simulink model "BaseEngineController_A02_bak"  (Simulink 7.7)
settings: Solver=FixedStepDiscrete  StartTime=0.0  StopTime=10.0  RelTol=1e-3
PreLoadFcn:
  | try, eval([bdroot '_setup']); catch, eval('model_setup', ''); end
InitFcn:
  | try, eval([bdroot '_setup']); catch, eval('model_setup', ''); end
undefined symbols (not defined in the file or in its folder): ECU555, High, motohawk_build_version, motohawk_get_faults, motohawk_trunc_name, motohawk_vardec_config_group, motohawk_vardec_path, True
libraries: MotoHawk_lib x1145, AutoDoc_lib x786, motohawk_control_primitives_lib x114, motohawk_engine_lib x19, motohawk_annotations_lib x12, simulink x11, simulink_extras x1
(part 23 of 23)

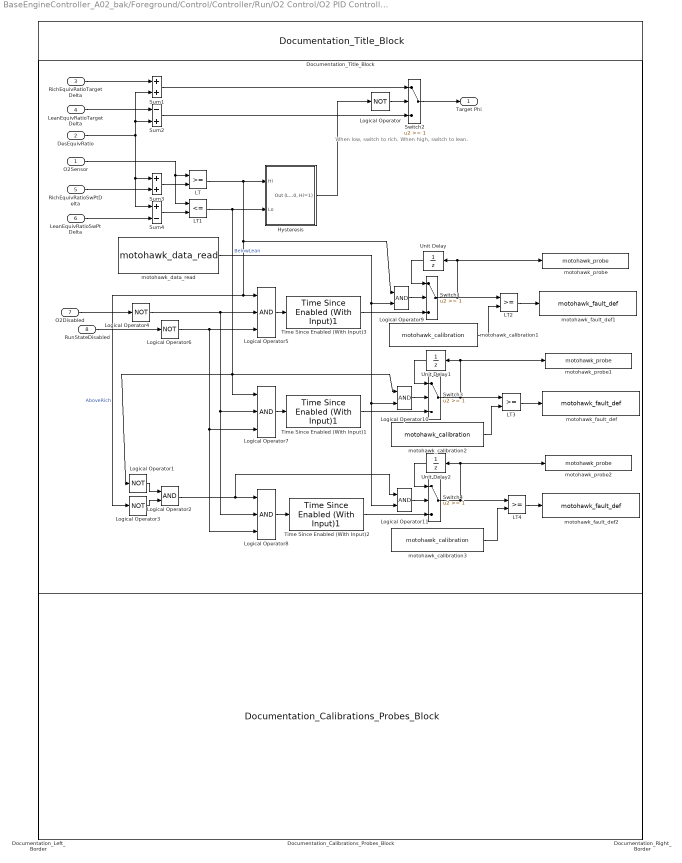
== system BaseEngineController_A02_bak/Foreground/Control/Controller/Run/O2 Control/O2 PID Controller/Equivalence Ratio Perturbation ==
  note: When low, switch to rich.
      | When high, switch to lean.
  O2Sensor : Inport
  DesEquivRatio : Inport  Port=2
  RichEquivRatioTargetDelta : Inport  Port=3
  LeanEquivRatioTargetDelta : Inport  Port=4
  RichEquivRatioSwPtDelta : Inport  Port=5
  LeanEquivRatioSwPtDelta : Inport  Port=6
  O2Disabled : Inport  Port=7
  RunStateDisabled : Inport  Port=8
  Documentation_Calibrations_Probes_Block : AutoDoc_lib/Documentation_Calibrations_Probes_Block
  Documentation_Left_Border : AutoDoc_lib/Documentation_Left_Border  layout=Portrait
  Documentation_Right_Border : AutoDoc_lib/Documentation_Right_Border  layout=Portrait
  Documentation_Title_Block : AutoDoc_lib/Documentation_Title_Block
  Hysteresis : Subsystem
  LT{,2..4} (4 alike) : RelationalOperator  ZeroCross=off  InputSameDT=on
  LT1 : RelationalOperator  Operator=<=  ZeroCross=off  InputSameDT=on
  Logical Operator{,1,3..4,6} (5 alike) : Logic  Operator=NOT
  Logical Operator{2,9..11} (4 alike) : Logic
  Logical Operator5 : Logic  Inputs=3
  Logical Operator7 : Logic  Inputs=3
  Logical Operator8 : Logic  Inputs=3
  Sum1 : Sum  SaturateOnIntegerOverflow=on
  Sum2 : Sum  Inputs=-+  SaturateOnIntegerOverflow=on
  Sum3 : Sum  SaturateOnIntegerOverflow=on
  Sum4 : Sum  Inputs=+-  SaturateOnIntegerOverflow=on
  Switch{1..4} (4 alike) : Switch  Threshold=1  ZeroCross=off  InputSameDT=on
  Time Since Enabled (With Input)1 : motohawk_control_primitives_lib/Time Since Enabled (With Input)1  SystemSampleTime=-1  nam='O2LeanTimer'  samp_time=-1  init=0  upperlim=16000
  Time Since Enabled (With Input)2 : motohawk_control_primitives_lib/Time Since Enabled (With Input)1  SystemSampleTime=-1  nam='O2ActiveTimer'  samp_time=-1  init=0  upperlim=16000
  Time Since Enabled (With Input)3 : motohawk_control_primitives_lib/Time Since Enabled (With Input)1  SystemSampleTime=-1  nam='O2RichTimer'  samp_time=-1  init=0  upperlim=16000
  Unit Delay : UnitDelay  SampleTime=-1
  Unit Delay1 : UnitDelay  SampleTime=-1
  Unit Delay2 : UnitDelay  SampleTime=-1
  motohawk_calibration1 : MotoHawk_lib/Calibration & Probing Blocks/motohawk_calibration  nam='O2RichTimeThresh'  val=40  storage=Calibration  show_more=on  name_source=Use Parameter  data_type=Inherit from 'Default Value'  read_level=1  write_level=1  ovr_cals=on  view_as=Number  enum_struct={'Disabled', 'Enabled'}  vector_style=Wide Row  use_help_units=on  help='Time limit the O2 feedback can sit abovethe rich switch point before flagging O2FailedRich fault'  units='s'  use_header_enum=off  row_header_enum={}  col_header_enum={}  use_min_max=off  min_val=-inf  max_val=inf  use_precision=off  precision=''  gain=1  offset=0  exp=1  use_mototune_group=on  mototune_group='Diagnostics & Protection | Fault Thresholds'
  motohawk_calibration2 : MotoHawk_lib/Calibration & Probing Blocks/motohawk_calibration  nam='O2LeanTimeThresh'  val=10  storage=Calibration  show_more=on  name_source=Use Parameter  data_type=Inherit from 'Default Value'  read_level=1  write_level=1  ovr_cals=on  view_as=Number  enum_struct={'Disabled', 'Enabled'}  vector_style=Wide Row  use_help_units=on  help='Time limit the O2 feedback can sit below the lean switch point before flagging O2FailedLean fault'  units='s'  use_header_enum=off  row_header_enum={}  col_header_enum={}  use_min_max=off  min_val=-inf  max_val=inf  use_precision=off  precision=''  gain=1  offset=0  exp=1  use_mototune_group=on  mototune_group='Diagnostics & Protection | Fault Thresholds'
  motohawk_calibration3 : MotoHawk_lib/Calibration & Probing Blocks/motohawk_calibration  nam='O2ActiveTimeThresh'  val=40  storage=Calibration  show_more=on  name_source=Use Parameter  data_type=Inherit from 'Default Value'  read_level=1  write_level=1  ovr_cals=on  view_as=Number  enum_struct={'Disabled', 'Enabled'}  vector_style=Wide Row  use_help_units=on  help='Time limit O2 feedback can sit between rich and lean switch points before flagging O2NotActive fault'  units='s'  use_header_enum=off  row_header_enum={}  col_header_enum={}  use_min_max=off  min_val=-inf  max_val=inf  use_precision=off  precision=''  gain=1  offset=0  exp=1  use_mototune_group=on  mototune_group='Diagnostics & Protection | Fault Thresholds'
  motohawk_data_read : MotoHawk_lib/Data Storage Blocks/motohawk_data_read  source=Lookup By Name  structure=Scalar  operation=Read scalar from location by index  sz_vec=4  sz_row=2  sz_col=3  typ=boolean  nam='DisableO2FaultAction'  checkNull=on  struct_inst_name='Struct1'  struct_nam='MyStruct'
  motohawk_fault_def : MotoHawk_lib/Fault Management Blocks/motohawk_fault_def  nam='O2FailedLean'  mode=Enabled  id=[]  x=25  y=50  input_suspected=on  indeterminate=off  increment_xy=on  downsample_count=1  ovr_cals=on  ovr_actions=on  show_actions=off  action1_name=''  action1_cond=(None)  action2_name=''  action2_cond=(None)  action3_name=''  action3_cond=(None)  action4_name=''  action4_cond=(None)
  motohawk_fault_def1 : MotoHawk_lib/Fault Management Blocks/motohawk_fault_def  nam='O2FailedRich'  mode=Enabled  id=[]  x=25  y=50  input_suspected=on  indeterminate=off  increment_xy=on  downsample_count=1  ovr_cals=on  ovr_actions=on  show_actions=off  action1_name=''  action1_cond=(None)  action2_name=''  action2_cond=(None)  action3_name=''  action3_cond=(None)  action4_name=''  action4_cond=(None)
  motohawk_fault_def2 : MotoHawk_lib/Fault Management Blocks/motohawk_fault_def  nam='O2NotActive'  mode=Enabled  id=[]  x=25  y=50  input_suspected=on  indeterminate=off  increment_xy=on  downsample_count=1  ovr_cals=on  ovr_actions=on  show_actions=off  action1_name=''  action1_cond=(None)  action2_name=''  action2_cond=(None)  action3_name=''  action3_cond=(None)  action4_name=''  action4_cond=(None)
  motohawk_probe : MotoHawk_lib/Calibration & Probing Blocks/motohawk_probe  nam='O2RichTime'  show_more=on  name_source=Use Parameter  read_level=1  view_as=Number  enum_struct={'Disabled', 'Enabled'}  vector_style=Wide Row  use_help_units=off  help=''  units=''  use_header_enum=off  row_header_enum={}  col_header_enum={}  use_precision=off  precision=''  gain=1  offset=0  exp=1  use_mototune_group=on  mototune_group='Diagnostics & Protection | Fault Thresholds'
  motohawk_probe1 : MotoHawk_lib/Calibration & Probing Blocks/motohawk_probe  nam='O2LeanTime'  show_more=on  name_source=Use Parameter  read_level=1  view_as=Number  enum_struct={'Disabled', 'Enabled'}  vector_style=Wide Row  use_help_units=off  help=''  units=''  use_header_enum=off  row_header_enum={}  col_header_enum={}  use_precision=off  precision=''  gain=1  offset=0  exp=1  use_mototune_group=on  mototune_group='Diagnostics & Protection | Fault Thresholds'
  motohawk_probe2 : MotoHawk_lib/Calibration & Probing Blocks/motohawk_probe  nam='O2NotActiveTime'  show_more=on  name_source=Use Parameter  read_level=1  view_as=Number  enum_struct={'Disabled', 'Enabled'}  vector_style=Wide Row  use_help_units=off  help=''  units=''  use_header_enum=off  row_header_enum={}  col_header_enum={}  use_precision=off  precision=''  gain=1  offset=0  exp=1  use_mototune_group=on  mototune_group='Diagnostics & Protection | Fault Thresholds'
  Target Phi : Outport
  wires:
  Sum3/1 -> LT/2
  Sum4/1 -> LT1/2
  Switch2/1 -> Target Phi/1
  DesEquivRatio/1 -> Sum1/2, Sum2/2, Sum4/1, Sum3/1
  RichEquivRatioSwPtDelta/1 -> Sum3/2
  LeanEquivRatioSwPtDelta/1 -> Sum4/2
  Sum2/1 -> Switch2/3
  Sum1/1 -> Switch2/1
  LeanEquivRatioTargetDelta/1 -> Sum2/1
  RichEquivRatioTargetDelta/1 -> Sum1/1
  LT/1 -> Hysteresis/1(Hi), Logical Operator5/1, Logical Operator3/1, Logical Operator9/1  "AboveRich"
  LT1/1 -> Hysteresis/2(Lo), Logical Operator1/1, Logical Operator7/1, Logical Operator10/1  "BelowLean"
  Hysteresis/1("Out (Lo=0, Hi=1)") -> Logical Operator/1
  O2Sensor/1 -> LT1/1, LT/1
  Logical Operator/1 -> Switch2/2
  motohawk_calibration1/1 -> LT2/2
  LT2/1 -> motohawk_fault_def1/1
  LT3/1 -> motohawk_fault_def/1
  motohawk_calibration2/1 -> LT3/2
  LT4/1 -> motohawk_fault_def2/1
  motohawk_calibration3/1 -> LT4/2
  Logical Operator1/1 -> Logical Operator2/1
  Logical Operator3/1 -> Logical Operator2/2
  Logical Operator4/1 -> Logical Operator5/2, Logical Operator7/2, Logical Operator8/2
  O2Disabled/1 -> Logical Operator4/1
  RunStateDisabled/1 -> Logical Operator6/1
  Logical Operator6/1 -> Logical Operator5/3, Logical Operator7/3, Logical Operator8/3
  Logical Operator2/1 -> Logical Operator8/1, Logical Operator11/1
  Logical Operator5/1 -> Time Since Enabled (With Input)3/1
  Logical Operator7/1 -> Time Since Enabled (With Input)1/1
  Logical Operator8/1 -> Time Since Enabled (With Input)2/1
  Unit Delay/1 -> Switch1/1
  Time Since Enabled (With Input)3/1 -> Switch1/3
  Switch1/1 -> LT2/1, Unit Delay/1, motohawk_probe/1
  Logical Operator9/1 -> Switch1/2
  motohawk_data_read/1 -> Logical Operator9/2, Logical Operator10/2, Logical Operator11/2
  Unit Delay1/1 -> Switch3/1
  Switch3/1 -> LT3/1, Unit Delay1/1, motohawk_probe1/1
  Logical Operator10/1 -> Switch3/2
  Time Since Enabled (With Input)1/1 -> Switch3/3
  Unit Delay2/1 -> Switch4/1
  Switch4/1 -> LT4/1, Unit Delay2/1, motohawk_probe2/1
  Logical Operator11/1 -> Switch4/2
  Time Since Enabled (With Input)2/1 -> Switch4/3

== system BaseEngineController_A02_bak/Foreground/Control/Diagnostics/Service Intervals/Run Timers/hour meter/motohawk_override_abs ==
  note: MotoHawk Absolute Override
  In1 : Inport
  Data Type Conversion : DataTypeConversion
  Gain : Gain  Gain=3600
  If : If  IfExpression=u1 ~= 0
  Merge : Merge
  NewValue : Subsystem  TreatAsAtomicUnit=on
  OldValue : Subsystem  TreatAsAtomicUnit=on
  new_value : MotoHawk_lib/Calibration & Probing Blocks/motohawk_calibration  nam=[parent_name '_new']  val=0  storage=Display  show_more=on  name_source=Use Parameter  data_type=Inherit via back propagation  read_level=1  write_level=1  ovr_cals=on  view_as=Number  enum_struct=enum_struct  vector_style=Wide Row  use_help_units=on  help=parent_help  units=parent_units  use_header_enum=off  row_header_enum={}  col_header_enum={}  use_min_max=on  min_val=parent_min  max_val=parent_max  use_precision=on  precision=parent_precision  gain=parent_gain  offset=parent_offset  exp=parent_exp  use_mototune_group=on  mototune_group=group
  override_enable : MotoHawk_lib/Calibration & Probing Blocks/motohawk_calibration  nam=[parent_name '_ovr']  val=false  storage=Display  show_more=on  name_source=Use Parameter  data_type=boolean  read_level=1  write_level=1  ovr_cals=on  view_as=Enumeration  enum_struct={'Pass-Through', 'Override'}  vector_style=Wide Row  use_help_units=off  help=''  units=''  use_header_enum=off  row_header_enum={}  col_header_enum={}  use_min_max=off  min_val=-inf  max_val=inf  use_precision=off  precision=''  gain=1  offset=0  exp=1  use_mototune_group=on  mototune_group=group
  Out1 : Outport
  wires:
  In1/1 -> OldValue/1(In1)
  Merge/1 -> Out1/1
  NewValue/1(Out1) -> Merge/1
  OldValue/1(Out1) -> Merge/2
  override_enable/1 -> If/1
  If/1 -> NewValue/Action
  If/2 -> OldValue/Action
  new_value/1 -> Gain/1
  Data Type Conversion/1 -> NewValue/1(In1)
  Gain/1 -> Data Type Conversion/1

== system BaseEngineController_A02_bak/Foreground/Control/VirtualSensors/LoadBasedCalcs/Normalized Effective Combustion Chamber Temperature/Integrate Offset To ECT/Integrator ==
  note: Discrete Integrator,
      | with output and state saturation.
  In : Inport
  Rst : Inport  Port=2
  IC : Inport  Port=3
  Min : Inport  Port=4
  Max : Inport  Port=5
  Data Type Conversion : DataTypeConversion  RndMeth=Zero
  Product : Product  SaturateOnIntegerOverflow=on  InputSameDT=on  OutDataTypeStr=Inherit: Same as first input
  Saturation : Subsystem  mask(Saturation){}
      mask help: Saturation block.
      mask Display:
      | patch([0 0 1 1], [0 0.075 0.075 0], [230/255 0/255 0/255])
      | patch([0 0 1 1], [0.025 0.05 0.05 0.025], [150/255 0/255 0/255])
      | patch([0 0 1 1], [0 0.025 0.025 0], [0/255 0/255 0/255])
      | 
      | plot([.2 .4 .6 .8], [.2 .2 .9 .9])
  Sum : Sum  Inputs=++|  SaturateOnIntegerOverflow=on  InputSameDT=on
  Switch1 : Switch  Threshold=1  ZeroCross=off  SaturateOnIntegerOverflow=on  InputSameDT=on
  Unit Delay : UnitDelay  SampleTime=-1
  motohawk_delta_time1 : MotoHawk_lib/Extra Development Blocks/motohawk_delta_time  samp_time=-1  init_val=0  mode=Seconds (double)
  Out : Outport
  wires:
  Saturation/1(out) -> Unit Delay/1
  Sum/1 -> Saturation/1(in)
  Switch1/1 -> Sum/1, Out/1
  motohawk_delta_time1/1 -> Product/2
  In/1 -> Product/1
  Product/1 -> Sum/2
  Unit Delay/1 -> Switch1/3
  Rst/1 -> Data Type Conversion/1
  Data Type Conversion/1 -> Switch1/2
  Min/1 -> Saturation/2(min)
  Max/1 -> Saturation/3(max)
  IC/1 -> Switch1/1

== system BaseEngineController_A02_bak/Foreground/Control/Controller/Run/Fuel System Manager/Fuel Calculation/Calculate Fuel Flow/Calculate A/F Ratio ==
  Ethanol_xx [%] : Inport
  " " : Constant  Value=1.0
  Add : Sum
  Add1 : Sum  Inputs=+-
  Documentation_Calibrations_Probes_Block : AutoDoc_lib/Documentation_Calibrations_Probes_Block
  Documentation_Left_Border : AutoDoc_lib/Documentation_Left_Border  layout=Portrait
  Documentation_Right_Border : AutoDoc_lib/Documentation_Right_Border  layout=Portrait
  Documentation_Title_Block : AutoDoc_lib/Documentation_Title_Block
  Gain : Gain  Gain=.01
  Product1 : Product  RndMeth=Zero
  Product2 : Product  RndMeth=Zero
  motohawk_calibration2 : MotoHawk_lib/Calibration & Probing Blocks/motohawk_calibration  nam='AF_Gasoline'  val=14.6  storage=Calibration  show_more=on  name_source=Use Parameter  data_type=Inherit from 'Default Value'  read_level=1  write_level=1  ovr_cals=on  view_as=Number  enum_struct={'Disabled', 'Enabled'}  vector_style=Wide Row  use_help_units=on  help='Stoichiometric ratio for gasoline'  units='mult'  use_header_enum=off  row_header_enum={}  col_header_enum={}  use_min_max=on  min_val=5  max_val=30  use_precision=off  precision=''  gain=1  offset=0  exp=1  use_mototune_group=on  mototune_group=motohawk_vardec_path(gcb)
  motohawk_calibration3 : MotoHawk_lib/Calibration & Probing Blocks/motohawk_calibration  nam='AF_Ethanol'  val=9.0  storage=Calibration  show_more=on  name_source=Use Parameter  data_type=Inherit from 'Default Value'  read_level=1  write_level=1  ovr_cals=on  view_as=Number  enum_struct={'Disabled', 'Enabled'}  vector_style=Wide Row  use_help_units=on  help='Stoichiometric ratio for ethanol'  units='mult'  use_header_enum=off  row_header_enum={}  col_header_enum={}  use_min_max=on  min_val=5  max_val=30  use_precision=off  precision=''  gain=1  offset=0  exp=1  use_mototune_group=on  mototune_group=motohawk_vardec_path(gcb)
  Desired A/F Ratio : Outport
  wires:
  motohawk_calibration3/1 -> Product2/1
  motohawk_calibration2/1 -> Product1/1
  Product1/1 -> Add/1
  Product2/1 -> Add/2
  Add/1 -> Desired A/F Ratio/1
  Gain/1 -> Product2/2, Add1/2
  Add1/1 -> Product1/2
  " "/1 -> Add1/1
  Ethanol_xx [%]/1 -> Gain/1

== system BaseEngineController_A02_bak/Foreground/Control/Controller/Run/Fuel System Manager/Injector Manager/Delta TPS Transient Fueling/ECT Transient Fueling ==
  ECTIdx : Inport
  Enable : EnablePort
  Documentation_Calibrations_Probes_Block : AutoDoc_lib/Documentation_Calibrations_Probes_Block
  Documentation_Left_Border : AutoDoc_lib/Documentation_Left_Border  layout=Portrait
  Documentation_Right_Border : AutoDoc_lib/Documentation_Right_Border  layout=Portrait
  Documentation_Title_Block : AutoDoc_lib/Documentation_Title_Block
  motohawk_interpolation_1d : MotoHawk_lib/Lookup Tables/motohawk_interpolation_1d  nam='ECTTransFuelMult'  table_data=[1 1 1 1 1]  show_more=on  data_type=Inherit from 'Table Data'  storage=NonAdaptive  read_level=1  write_level=1  ovr_cals=on  use_help=on  help="'Transient fuel multiplier based on ECT.  Only engaged during transient fueling'"  units=''  use_min_max=off  min_val=-inf  max_val=inf  use_precision=off  precision=''  gain=1  offset=0  exp=1  use_links=off  prelookup_block=''  output_name=''  use_mototune_group=on  mototune_group=motohawk_vardec_path(gcb)
  Mult : Outport  OutputWhenDisabled=reset  InitialOutput=1
  wires:
  motohawk_interpolation_1d/1 -> Mult/1
  ECTIdx/1 -> motohawk_interpolation_1d/1

== system BaseEngineController_A02_bak/Foreground/Control/Controller/Run/Fuel System Manager/Injector Manager/Tau-X Transient Fueling/Enabled Tau-X Algorithm ==
  note: Calculate fuel vaporization factor.
      | Fuel evaporation is linear with respect to this term.
      | FilmTemp / ln(ManifoldPressure)
  MAF [g/s] : Inport
  IAT [degC] : Inport  Port=2
  ECT [degC] : Inport  Port=3
  MAP [kPa] : Inport  Port=4
  Fdes [g/s] : Inport  Port=5
  Action Port : ActionPort  InitializeStates=reset  ActionType=then
  Add : Sum
  Add1 : Sum
  Cals_and_Probes_Page : AutoDoc_lib/Cals_and_Probes_Page  SystemSampleTime=-1
  Constant : Constant  Value=273.15
  Documentation_Calibrations_Probes_Block : AutoDoc_lib/Documentation_Calibrations_Probes_Block
  Documentation_Left_Border : AutoDoc_lib/Documentation_Left_Border  layout=Portrait
  Documentation_Right_Border : AutoDoc_lib/Documentation_Right_Border  layout=Portrait
  Documentation_Title_Block : AutoDoc_lib/Documentation_Title_Block
  Film Temperature Average of ECT and IAT : Fcn  Expr=(u(1)*u(3)/100 + u(2)*(1-u(3)/100))
  Math Function : Math  Operator=log  SaturateOnIntegerOverflow=on
  Mux : Mux  Inputs=3
  Product : Product  Inputs=*/  SaturateOnIntegerOverflow=on  InputSameDT=on  OutDataTypeStr=Inherit: Same as first input
  Saturation : Saturate  UpperLimit=inf  LowerLimit=0
  Saturation1 : Saturate  UpperLimit=inf  LowerLimit=.0001
  Saturation2 : Saturate  UpperLimit=inf  LowerLimit=.0001
  Tau-X Fuel Model : Subsystem  mask{samp_time=-1}
      mask prompts: samp_time "Sample Time"
      mask Display:
      | patch([0 0 1 1], 0.5*[0 0.075 0.075 0], [230/255 0/255 0/255])
      | patch([0 0 1 1], 0.5*[0.025 0.05 0.05 0.025], [150/255 0/255 0/255])
      | patch([0 0 1 1], 0.5*[0 0.025 0.025 0], [0/255 0/255 0/255])
      | 
      | disp('TRANSIENT FUELING\nTAU-X MODEL');
  Zero-Order Hold : ZeroOrderHold  SampleTime=-1
  motohawk_calibration1 : MotoHawk_lib/Calibration & Probing Blocks/motohawk_calibration  nam='FuelVaporFactorBias'  val=50  storage=Calibration  show_more=on  name_source=Use Parameter  data_type=Inherit from 'Default Value'  read_level=1  write_level=1  ovr_cals=on  view_as=Number  enum_struct={'Disabled', 'Enabled'}  vector_style=Wide Row  use_help_units=on  help="'Percent bias between contribution of IAT and ECT to FuelVaporFactor.  100% is all IAT.'"  units='%'  use_header_enum=off  row_header_enum={}  col_header_enum={}  use_min_max=on  min_val=0  max_val=100  use_precision=off  precision=''  gain=1  offset=0  exp=1  use_mototune_group=on  mototune_group=motohawk_vardec_path(gcb)
  motohawk_calibration2 : MotoHawk_lib/Calibration & Probing Blocks/motohawk_calibration  nam='TransientFuelMaxPuddle'  val=1000  storage=Calibration  show_more=on  name_source=Use Parameter  data_type=Inherit from 'Default Value'  read_level=1  write_level=1  ovr_cals=on  view_as=Number  enum_struct={'Disabled', 'Enabled'}  vector_style=Wide Row  use_help_units=on  help='Maximum fuel puddle size'  units='mg'  use_header_enum=off  row_header_enum={}  col_header_enum={}  use_min_max=on  min_val=0  max_val=inf  use_precision=off  precision=''  gain=1  offset=0  exp=1  use_mototune_group=on  mototune_group=motohawk_vardec_path(gcb)
  motohawk_calibration3 : MotoHawk_lib/Calibration & Probing Blocks/motohawk_calibration  nam='TransientFuelInitPuddle'  val=20  storage=Calibration  show_more=on  name_source=Use Parameter  data_type=Inherit from 'Default Value'  read_level=1  write_level=1  ovr_cals=on  view_as=Number  enum_struct={'Disabled', 'Enabled'}  vector_style=Wide Row  use_help_units=on  help='Initial fuel puddle size'  units='mg'  use_header_enum=off  row_header_enum={}  col_header_enum={}  use_min_max=on  min_val=0  max_val=inf  use_precision=off  precision=''  gain=1  offset=0  exp=1  use_mototune_group=on  mototune_group=motohawk_vardec_path(gcb)
  motohawk_interpolation_2d1 : MotoHawk_lib/Lookup Tables/motohawk_interpolation_2d  nam='TransientFuelTau'  table_data=[1 0.87 0.74 0.61 0.5;0.87 0.78 0.65 0.51 0.4;0.74 0.65 0.56 0.43 0.3;0.61 0.51 0.43 0.36 0.2;0.5 0.4 0.3 0.2 0.1]  show_more=on  data_type=Inherit from 'Table Data'  storage=NonAdaptive  read_level=1  write_level=1  ovr_cals=on  use_help=on  help='Fuel evaporation time constant'  units='sec'  use_min_max=on  min_val=0  max_val=inf  use_precision=off  precision=''  gain=1  offset=0  exp=1  use_links=off  row_prelookup_block=''  col_prelookup_block=''  output_name=''  use_mototune_group=on  mototune_group=motohawk_vardec_path(gcb)
  motohawk_interpolation_2d2 : MotoHawk_lib/Lookup Tables/motohawk_interpolation_2d  nam='TransientFuelX'  table_data=[0.6016 0.5234 0.4531 0.375 0.2969;0.5547 0.4844 0.4141 0.3438 0.2734;0.5 0.4375 0.3828 0.3125 0.25;0.4531 0.3984 0.3438 0.2813 0.2266;0.3984 0.3516 0.3047 0.25 0.2031]  show_more=on  data_type=Inherit from 'Table Data'  storage=NonAdaptive  read_level=1  write_level=1  ovr_cals=on  use_help=on  help='Fraction of injected fuel to enter the puddle'  units='frac'  use_min_max=on  min_val=0  max_val=.999  use_precision=off  precision=''  gain=1  offset=0  exp=1  use_links=off  row_prelookup_block=''  col_prelookup_block=''  output_name=''  use_mototune_group=on  mototune_group=motohawk_vardec_path(gcb)
  motohawk_prelookup2 : MotoHawk_lib/Lookup Tables/motohawk_prelookup  nam='FuelVapFactor'  breakpoint_data=[-5 2.5 10 17.5 25]  show_more=on  data_type=Inherit from 'Table Data'  storage=Constant  read_level=1  write_level=1  ovr_cals=on  use_help=on  help='Breakpoints for fuel vaporization factor'  units='degC/log(kPa)'  use_min_max=off  min_val=-inf  max_val=inf  use_precision=off  precision=''  gain=1  offset=0  exp=1  use_links=on  input_name=''  use_mototune_group=on  mototune_group=motohawk_vardec_path(gcb)
  motohawk_prelookup3 : MotoHawk_lib/Lookup Tables/motohawk_prelookup  nam='MAF05'  breakpoint_data=[2 4 8 16 32]  show_more=on  data_type=Inherit from 'Table Data'  storage=Constant  read_level=1  write_level=1  ovr_cals=on  use_help=on  help='Breakpoints for Mass Airflow'  units='g/s'  use_min_max=off  min_val=-inf  max_val=inf  use_precision=off  precision=''  gain=1  offset=0  exp=1  use_links=on  input_name=''  use_mototune_group=on  mototune_group=motohawk_vardec_path(gcb)
  motohawk_probe1 : MotoHawk_lib/Calibration & Probing Blocks/motohawk_probe  nam='TransientFuelPuddleMass'  show_more=on  name_source=Use Parameter  read_level=1  view_as=Number  enum_struct={'Disabled', 'Enabled'}  vector_style=Wide Row  use_help_units=on  help='Current modeled fuel puddle mass for Tau-X transient fuel'  units='mg'  use_header_enum=off  row_header_enum={}  col_header_enum={}  use_precision=off  precision=''  gain=1  offset=0  exp=1  use_mototune_group=on  mototune_group=motohawk_vardec_path(gcb)
  motohawk_probe2 : MotoHawk_lib/Calibration & Probing Blocks/motohawk_probe  nam='FuelVapFactor'  show_more=on  name_source=Use Parameter  read_level=1  view_as=Number  enum_struct={'Disabled', 'Enabled'}  vector_style=Wide Row  use_help_units=on  help='Fuel vaporization factor - Fuel evaporation is linear with respect to this term'  units='degC/log(kPa)'  use_header_enum=off  row_header_enum={}  col_header_enum={}  use_precision=off  precision=''  gain=1  offset=0  exp=1  use_mototune_group=on  mototune_group=motohawk_vardec_path(gcb)
  motohawk_probe3 : MotoHawk_lib/Calibration & Probing Blocks/motohawk_probe  nam='TransientFuel_X_Mult'  show_more=on  name_source=Use Parameter  read_level=1  view_as=Number  enum_struct={'Disabled', 'Enabled'}  vector_style=Wide Row  use_help_units=on  help='Multiplier on desired fuel flow due to X contribution (1/(1-X)).'  units=''  use_header_enum=off  row_header_enum={}  col_header_enum={}  use_precision=off  precision=''  gain=1  offset=0  exp=1  use_mototune_group=on  mototune_group=motohawk_vardec_path(gcb)
  motohawk_probe4 : MotoHawk_lib/Calibration & Probing Blocks/motohawk_probe  nam='TransientFuel_Tau_Ofst'  show_more=on  name_source=Use Parameter  read_level=1  view_as=Number  enum_struct={'Disabled', 'Enabled'}  vector_style=Wide Row  use_help_units=on  help='Offset applied to desired fuel flow based on Tau contribution (-M_pud/Tau).'  units='g/s'  use_header_enum=off  row_header_enum={}  col_header_enum={}  use_precision=off  precision=''  gain=1  offset=0  exp=1  use_mototune_group=on  mototune_group=motohawk_vardec_path(gcb)
  motohawk_probe5 : MotoHawk_lib/Calibration & Probing Blocks/motohawk_probe  nam='TransientFuel_Total_Mult'  show_more=on  name_source=Use Parameter  read_level=1  view_as=Number  enum_struct={'Disabled', 'Enabled'}  vector_style=Wide Row  use_help_units=on  help='Total transient fuel multiplier applied to desired fuel flow resulting from Tau-X model.'  units=''  use_header_enum=off  row_header_enum={}  col_header_enum={}  use_precision=off  precision=''  gain=1  offset=0  exp=1  use_mototune_group=on  mototune_group=motohawk_vardec_path(gcb)
  motohawk_probe6 : MotoHawk_lib/Calibration & Probing Blocks/motohawk_probe  nam='TransientFuel_FuelRate'  show_more=on  name_source=Use Parameter  read_level=1  view_as=Number  enum_struct={'Disabled', 'Enabled'}  vector_style=Wide Row  use_help_units=on  help='Actual fuel rate applied to engine'  units='g/s'  use_header_enum=off  row_header_enum={}  col_header_enum={}  use_precision=off  precision=''  gain=1  offset=0  exp=1  use_mototune_group=on  mototune_group=motohawk_vardec_path(gcb)
  Finj [g/s] : Outport
  wires:
  motohawk_interpolation_2d2/1 -> Tau-X Fuel Model/2(X [frac])
  motohawk_interpolation_2d1/1 -> Tau-X Fuel Model/3(Tau [1/s])
  motohawk_prelookup2/1 -> motohawk_interpolation_2d1/2, motohawk_interpolation_2d2/2
  Zero-Order Hold/1 -> Finj [g/s]/1
  Tau-X Fuel Model/1(Finj [g/s]) -> Zero-Order Hold/1, motohawk_probe6/1
  MAP [kPa]/1 -> Saturation/1
  MAF [g/s]/1 -> motohawk_prelookup3/1
  Fdes [g/s]/1 -> Saturation2/1
  motohawk_prelookup3/1 -> motohawk_interpolation_2d1/1, motohawk_interpolation_2d2/1
  Product/1 -> motohawk_probe2/1, motohawk_prelookup2/1
  Math Function/1 -> Saturation1/1
  Film Temperature Average of ECT and IAT/1 -> Product/1
  Mux/1 -> Film Temperature Average of ECT and IAT/1
  Tau-X Fuel Model/2(PuddleMass [mg]) -> motohawk_probe1/1
  motohawk_calibration3/1 -> Tau-X Fuel Model/5(InitPuddle [mg])
  motohawk_calibration2/1 -> Tau-X Fuel Model/4(MaxPuddle [mg])
  Tau-X Fuel Model/3(X_Mult) -> motohawk_probe3/1
  Tau-X Fuel Model/4(Tau_Ofst) -> motohawk_probe4/1
  Tau-X Fuel Model/5(TranFuelMult) -> motohawk_probe5/1
  motohawk_calibration1/1 -> Mux/3
  Add/1 -> Mux/2
  Add1/1 -> Mux/1
  ECT [degC]/1 -> Add/2
  IAT [degC]/1 -> Add1/2
  Constant/1 -> Add1/1, Add/1
  Saturation/1 -> Math Function/1
  Saturation1/1 -> Product/2
  Saturation2/1 -> Tau-X Fuel Model/1(Fdes [g/s])

== system BaseEngineController_A02_bak/Foreground/Control/Controller/Run/Fuel System Manager/Injector Manager/Tau-X Transient Fueling/Pass-Through ==
  note: Simply pass-through desired fuel as injected fuel
      | when transient fueling is disabled
  Fdes [g/s] : Inport
  Action Port : ActionPort  InitializeStates=reset  ActionType=else
  Finj [g/s] : Outport
  wires:
  Fdes [g/s]/1 -> Finj [g/s]/1

== system BaseEngineController_A02_bak/Foreground/Control/Controller/Run/O2 Control/O2 PID Controller/Equivalence Ratio Perturbation/Hysteresis ==
  note: Hi & !Lo => Hi
      | Lo & !Hi => Lo
      | Otherwise, Hold
  Hi : Inport
  Lo : Inport  Port=2
  Combinatorial  Logic : CombinatorialLogic  TruthTable=[0 0;0 1;1 1;0 0]
  Demux : Demux  Outputs=2
  Documentation_Calibrations_Probes_Block : AutoDoc_lib/Documentation_Calibrations_Probes_Block
  Documentation_Left_Border : AutoDoc_lib/Documentation_Left_Border  layout=Portrait
  Documentation_Right_Border : AutoDoc_lib/Documentation_Right_Border  layout=Portrait
  Documentation_Title_Block : AutoDoc_lib/Documentation_Title_Block
  Mux : Mux  Inputs=2
  Switch1 : Switch  Threshold=0.5  SaturateOnIntegerOverflow=on  InputSameDT=on
  Unit Delay : UnitDelay  SampleTime=-1
  "Out (Lo=0, Hi=1)" : Outport
  wires:
  Mux/1 -> Combinatorial  Logic/1
  Combinatorial  Logic/1 -> Demux/1
  Demux/1 -> Switch1/1
  Demux/2 -> Switch1/2
  Unit Delay/1 -> Switch1/3
  Switch1/1 -> Unit Delay/1, "Out (Lo=0, Hi=1)"/1
  Hi/1 -> Mux/1
  Lo/1 -> Mux/2

== system BaseEngineController_A02_bak/Foreground/Control/Diagnostics/Service Intervals/Run Timers/hour meter/motohawk_override_abs/NewValue ==
  In1 : Inport
  Action Port : ActionPort  ActionType=then
  motohawk_code_coverage : MotoHawk_lib/Extra Development Blocks/motohawk_code_coverage
  Out1 : Outport
  wires:
  In1/1 -> Out1/1

== system BaseEngineController_A02_bak/Foreground/Control/Diagnostics/Service Intervals/Run Timers/hour meter/motohawk_override_abs/OldValue ==
  In1 : Inport
  Action Port : ActionPort  ActionType=else
  motohawk_code_coverage : MotoHawk_lib/Extra Development Blocks/motohawk_code_coverage
  Out1 : Outport
  wires:
  In1/1 -> Out1/1

== system BaseEngineController_A02_bak/Foreground/Control/VirtualSensors/LoadBasedCalcs/Normalized Effective Combustion Chamber Temperature/Integrate Offset To ECT/Integrator/Saturation ==
  note: Output 'In' when between Min and Max,
      | and saturate against limits otherwise.
  in : Inport
  min : Inport  Port=2
  max : Inport  Port=3
  MinMax : MinMax  Function=max  Inputs=2  ZeroCross=off  SaturateOnIntegerOverflow=on  InputSameDT=on
  MinMax1 : MinMax  Inputs=2  ZeroCross=off  SaturateOnIntegerOverflow=on  InputSameDT=on
  out : Outport
  wires:
  MinMax/1 -> MinMax1/1
  in/1 -> MinMax/1
  min/1 -> MinMax/2
  max/1 -> MinMax1/2
  MinMax1/1 -> out/1

== system BaseEngineController_A02_bak/Foreground/Control/Controller/Run/Fuel System Manager/Injector Manager/Tau-X Transient Fueling/Enabled Tau-X Algorithm/Tau-X Fuel Model ==
  note: Puddle mass is modeled by integrating
      | the difference between the desired fuel rate
      | and the actual fuel rate
  note: Tau - Puddle evaporation time constant
      | X - Fraction of fuel injected that adds to the puddle
  Fdes [g/s] : Inport
  X [frac] : Inport  Port=2
  Tau [1/s] : Inport  Port=3
  MaxPuddle [mg] : Inport  Port=4
  InitPuddle [mg] : Inport  Port=5
  Can't Have Negative Puddle Mass1 : Constant  Value=0  OutDataTypeStr=Inherit: Inherit via back propagation
  Can't have negative puddle mass : Constant  Value=0  OutDataTypeStr=Inherit: Inherit via back propagation
  Can't inject negative fuel : MinMax  Function=max  Inputs=2  SaturateOnIntegerOverflow=on  InputSameDT=on
  Compute using grams : Gain  Gain=1/1000  SaturateOnIntegerOverflow=on  OutDataTypeStr=Inherit: Same as input  ParamDataTypeStr=Inherit: Same as input
  Documentation_Calibrations_Probes_Block : AutoDoc_lib/Documentation_Calibrations_Probes_Block
  Documentation_Left_Border : AutoDoc_lib/Documentation_Left_Border  layout=Portrait
  Documentation_Right_Border : AutoDoc_lib/Documentation_Right_Border  layout=Portrait
  Documentation_Title_Block : AutoDoc_lib/Documentation_Title_Block
  Initialize puddle mass on first sample : motohawk_control_primitives_lib/First Time  SystemSampleTime=-1
  Integrator : motohawk_control_primitives_lib/Integrator  SystemSampleTime=-1  samp_time=samp_time
  Product : Product  Inputs=*/  SaturateOnIntegerOverflow=on  OutDataTypeStr=Inherit: Same as first input
  Store and report in milligrams : Gain  Gain=1000  SaturateOnIntegerOverflow=on  OutDataTypeStr=Inherit: Same as input  ParamDataTypeStr=Inherit: Same as input
  Sum1 : Sum  Inputs=+-|  SaturateOnIntegerOverflow=on  InputSameDT=on
  TauX Eq : Subsystem
  Finj [g/s] : Outport
  PuddleMass [mg] : Outport  Port=2
  X_Mult : Outport  Port=3
  Tau_Ofst : Outport  Port=4
  TranFuelMult : Outport  Port=5
  wires:
  Store and report in milligrams/1 -> Integrator/1
  Compute using grams/1 -> TauX Eq/4(Mpud [g])
  Fdes [g/s]/1 -> TauX Eq/1(Fdes [g/s]), Sum1/2, Product/2
  Tau [1/s]/1 -> TauX Eq/3(Tau [1/s])
  X [frac]/1 -> TauX Eq/2(X [frac])
  Sum1/1 -> Store and report in milligrams/1
  Integrator/1 -> Compute using grams/1, PuddleMass [mg]/1
  MaxPuddle [mg]/1 -> Integrator/5
  Can't have negative puddle mass/1 -> Integrator/4
  Initialize puddle mass on first sample/1 -> Integrator/2
  InitPuddle [mg]/1 -> Integrator/3
  TauX Eq/1(Finj [g/s]) -> Can't inject negative fuel/2
  Can't inject negative fuel/1 -> Finj [g/s]/1, Sum1/1, Product/1
  Can't Have Negative Puddle Mass1/1 -> Can't inject negative fuel/1
  TauX Eq/2(X_Mult) -> X_Mult/1
  TauX Eq/3(Tau_Ofst) -> Tau_Ofst/1
  Product/1 -> TranFuelMult/1

== system BaseEngineController_A02_bak/Foreground/Control/Controller/Run/Fuel System Manager/Injector Manager/Tau-X Transient Fueling/Enabled Tau-X Algorithm/Tau-X Fuel Model/TauX Eq ==
  Fdes [g/s] : Inport
  X [frac] : Inport  Port=2
  Tau [1/s] : Inport  Port=3
  Mpud [g] : Inport  Port=4
  Constant : Constant
  Documentation_Calibrations_Probes_Block : AutoDoc_lib/Documentation_Calibrations_Probes_Block
  Documentation_Left_Border : AutoDoc_lib/Documentation_Left_Border  layout=Portrait
  Documentation_Right_Border : AutoDoc_lib/Documentation_Right_Border  layout=Portrait
  Documentation_Title_Block : AutoDoc_lib/Documentation_Title_Block
  Product : Product  Inputs=*/  SaturateOnIntegerOverflow=on  InputSameDT=on  OutDataTypeStr=Inherit: Same as first input
  Product1 : Product  Inputs=**  SaturateOnIntegerOverflow=on  InputSameDT=on  OutDataTypeStr=Inherit: Same as first input
  Product2 : Product  Inputs=*/  SaturateOnIntegerOverflow=on  InputSameDT=on  OutDataTypeStr=Inherit: Same as first input
  Sum : Sum  Inputs=|+-  SaturateOnIntegerOverflow=on  InputSameDT=on
  Sum1 : Sum  Inputs=|+-  SaturateOnIntegerOverflow=on  InputSameDT=on
  Finj [g/s] : Outport
  X_Mult : Outport  Port=2
  Tau_Ofst : Outport  Port=3
  wires:
  Product1/1 -> Finj [g/s]/1
  Product/1 -> Product1/2, X_Mult/1  "1 / (1 - X)"
  Sum1/1 -> Product1/1  "Fdes - Mpud / T"
  Product2/1 -> Sum1/2, Tau_Ofst/1
  Fdes [g/s]/1 -> Sum1/1
  Tau [1/s]/1 -> Product2/2
  Mpud [g]/1 -> Product2/1
  Sum/1 -> Product/2
  X [frac]/1 -> Sum/2
  Constant/1 -> Sum/1, Product/1

== stateflow chart Foreground/Control/ActuatorCharacterization/EGR Characterization/Signal Generator/Chart ==
  state default
    during: enout=1;
  state reset
    during: enout=0;
  state Init
    during: enout=0;
  reset -> default : [enout==0]
  default -> reset : [res==1]
  (default) -> Init
  Init -> default : [enin==1]
  data: enin input boolean; enout output double; res input boolean

== stateflow chart Foreground/Control/VirtualSensors/RawProcessed/EngineState/Engine State Machine ==
  settings: executeAtInitialization=1
  state Stall/
    en:State=1;
  state Run/
    en:State=3;
  state Crank/
    en:State=2;
  (default) -> Stall
  Stall -> Crank : [Crank]
  Crank -> Run [order 2] : [Run]
  Run -> Stall : [Stall]
  Crank -> Stall [order 1] : [Stall]
  data: Stall input boolean; Crank input boolean; Run input boolean; State output uint8

== stateflow chart Foreground/Control/Diagnostics/Freeze Frame Trigger/Freeze-Frame Trigger ==
  state FREEZE_FRAME_TRIGGER [group]
    state AT_STARTUP
      entry: FFIDs = FFIDsIn
      entry: FltOrder = FltOrderIn
      entry: FltOrderMax = FltOrderMaxIn
    state TIME_STEP
      entry: ActvFltCount = 0
      entry: FltOrderCount = 0
    state CHECK_FOR_ACTIVE_FAULTS [group]
      (junction 35) -> (junction 36) [order 1] : [ ActvFlts[ActvFltCount] == 0 ]
      (junction 36) -> (junction 35) [order 1] : [ ActvFltCount < NumFlts - 1 ]
          | { ActvFltCount = ...
          |   ActvFltCount + 1 }
    state CHECK_FOR_EMPTY_SLOT_OR_FAULT_EXISTING_IN_FF [group]
      (junction 39) -> (junction 38) [order 1] : [ FFIDs[FltOrderCount] ~= 0 && ...
          |   FFIDs[FltOrderCount] ~= ActvFltCount + 1 ]
      (junction 38) -> (junction 39) [order 1] : [ FltOrderCount < NumFF - 1 ]
          | { FltOrderCount = ...
          |   FltOrderCount + 1 }
    state REPLACE_OLDEST [group]
      (junction 44) -> (junction 43) [order 2]
      (junction 44) -> (junction 43) [order 1] : [ FltOrder[FFIDs[FltOrderCount]-1] < FltOrderOld ]
          | { FltOrderOld = FltOrder[FFIDs[FltOrderCount]-1]
          |   OldIndex = FltOrderCount }
      (junction 43) -> (junction 44) [order 1] : [ FltOrderCount < NumFF - 1 ]
          | { FltOrderCount = ...
          |   FltOrderCount + 1 }
    (default) -> AT_STARTUP
    AT_STARTUP -> (junction 35)
    (junction 39) -> (junction 37) [order 2] : { FFIDs[FltOrderCount] = ActvFltCount + 1
        |   FFTrigg[FltOrderCount] = 1 }
    (junction 36) -> TIME_STEP [order 2]
    TIME_STEP -> (junction 35) : { FFTrigg = FFTriggZeros }
    (junction 35) -> (junction 39) [order 2] : { FltOrderMax = FltOrderMax + 1
        |   FltOrder[ActvFltCount] = FltOrderMax
        |   FltOrderCount = 0 }
    (junction 38) -> (junction 44) [order 2] : { FltOrderCount = 0
        |   FltOrderOld = FltOrderMax }
    (junction 42) -> (junction 36)
    (junction 41) -> (junction 40)
    (junction 40) -> (junction 42)
    (junction 37) -> (junction 41)
    (junction 43) -> (junction 41) [order 2] : { FFIDs[OldIndex] = ActvFltCount + 1
        |   FFTrigg[OldIndex] = 1 }
  data: ActvFlts input double; FFTriggZeros input double; FFIDs output uint8; FFIDsIn input double; FFTrigg output boolean size=6; NumFF input double; FltOrderIn input double; NumFlts input uint8; FltOrderMaxIn input double; FltOrder output uint16; FltOrderMax output uint16; ActvFltCount local uint8; OldIndex local uint8; FltOrderCount local uint8; FltOrderOld local uint16

== stateflow chart Foreground/Control/Controller/Hybrid/Remy Control ==
  state default
    du: cranking=0;
    idle=0;
    APPRamp=0;
    torqueRamp=0;
    remyEn=0;
    maxRPM=0;
    motorTQ=0;
    generatingTQ=0;
  state cranking
    en: count=0;
    du: count++;
    cranking=1;
    idle=0;
    APPRamp=0;
    torqueRamp=0;
    remyEn=1;
    maxRPM=maxCrankRPM;
    motorTQ=crankTQ;
    generatingTQ=1;
  state startFail
    en: count=0;
    du: count++;
    cranking=0;
    idle=0;
    APPRamp=0;
    torqueRamp=0;
    remyEn=0;
    maxRPM=0;
    motorTQ=0;
    generatingTQ=0;
  state idle
    du: cranking=0;
    idle=1;
    APPRamp=0;
    torqueRamp=0;
    remyEn=1;
    maxRPM=maxMotorRPM;
    motorTQ=0;
    generatingTQ=0;
  state generating
    du: cranking=0;
    idle=0;
    APPRamp=1;
    torqueRamp=1;
    remyEn=1;
    maxRPM=maxMotorRPM;
    motorTQ=0;
    generatingTQ=1;
  default -> cranking : [genEnable]
  cranking -> startFail [order 1] : [count>=crankTime]
  cranking -> default [order 2] : [!genEnable]
  startFail -> default [order 1] : [!genEnable]
  startFail -> cranking [order 2] : [count>=crankWait]
  cranking -> idle [order 3] : [RPM>=caughtRPM]
  idle -> default [order 1] : [!genEnable]
  idle -> generating [order 2] : [genLoad]
  generating -> default [order 1] : [!genEnable]
  generating -> idle [order 2] : [!genLoad]
  (default) -> default
  data: genEnable input double; genLoad input double; RPM input double; crankTime input double; crankWait input double; caughtRPM input double; crankTQ input double; maxCrankRPM input double; maxMotorRPM input double; cranking output double; idle output double; APPRamp output double; torqueRamp output double; remyEn output double; maxRPM output double; motorTQ output double; generatingTQ output double; count local double

== stateflow chart Foreground/Control/Controller/Run/O2 Heater/Chart ==
  state Off
    du:HEATER=0;
  state On
    du:HEATER=1;
  (default) -> Off
  Off -> On : [NEECT<=(CAL-1)]
  On -> Off : [NEECT>=(CAL+1)]
  data: NEECT input double; CAL input double; HEATER output boolean

== matlab function Foreground/Control/Controller/Run/MaxGovernor/MaxGovState/MaxGOV Stateflow ==
  data: OffToOn input double; ETCMode output double; OnToTrans input double; TransToOn input double; TransToOff input double; State input double; Reset output double; Hold output double; StateO output double
function [ETCMode,Reset,Hold,StateO] = fcn(OffToOn,OnToTrans,TransToOn,TransToOff,State)

if (State == 0 && OffToOn == 1)
    StateO = 1;
elseif (State == 1 && OnToTrans == 1)
    StateO=2;
elseif (State == 2 && TransToOn == 1)
    StateO=1;
elseif (State == 2 && TransToOff == 1)
    StateO=0;
else
    StateO=State;
end

if State == 0
    ETCMode=0;
    Hold=0;
    Reset=0;
elseif State == 1;
    ETCMode=1;
    Hold=0;
    Reset=0;
elseif State == 2;
    ETCMode=0;
    Hold=1;
    Reset=0;
else
    ETCMode=0;
    Hold=0;
    Reset=0;
end

== stateflow chart Main Power Relay/Main Power Relay/ECUP Latch ==
  settings: executeAtInitialization=1
  state PowerDown/
    en:MPRDState=0;
  state PowerUp/
    en:MPRDState=1;
  (default) -> PowerDown
  PowerUp -> PowerDown : [!ECUP&&ShutDownTasks]
  PowerDown -> PowerUp : [ECUP]
  data: ECUP input boolean; ShutDownTasks input boolean; MPRDState output boolean

== stateflow chart Foreground/Control/ActuatorCharacterization/Electronic Throttle Controller/ETC Test  Manager ==
  settings: executeAtInitialization=1
  state PassThrough/
    en:DC_Override=0;
    en:TestComplete=0;
    en:SetpointMode=0;
    en:TestTime=0;
  state LowAdapt/
    en:DC_Override=0;
    en:TestComplete=0;
    en:SetpointMode=1;
    en:TestTime=0;
  state SpringTestSetpoint/
    en:DC_Override=0;
    en:TestComplete=0;
    en:SetpointMode=2;
    en:TestTime=0;
  state SpringTest/
    en:DC_Override=1;
    en:TestComplete=0;
    en:SetpointMode=0;
    en:TestTime=0;
    en:TimerOld=Timer;
  state SpringTestComplete/
    en:DC_Override=1;
    en:TestComplete=1;
    en:SetpointMode=0;
    en:TestTime=Timer-TimerOld;
  state ForcedShutdown/
    en:DC_Override=1;
    en:TestComplete=1;
    en:SetpointMode=0;
    en:TestTime=0;
  (default) -> PassThrough
  PassThrough -> LowAdapt : [ECUP_Off]
  LowAdapt -> SpringTestSetpoint [order 2] : [ECUP_Off&&LowAdaptError]
  SpringTestSetpoint -> SpringTest [order 2] : [ECUP_Off&&TPSHiThresh]
  SpringTest -> SpringTestComplete [order 2] : [ECUP_Off&&TPSLoThresh]
  LowAdapt -> PassThrough [order 3] : [!ECUP_Off]
  SpringTestSetpoint -> PassThrough [order 1] : [!ECUP_Off]
  SpringTestComplete -> PassThrough : [!ECUP_Off]
  SpringTest -> PassThrough [order 3] : [!ECUP_Off]
  SpringTest -> ForcedShutdown [order 1] : [Timer>5]
  LowAdapt -> ForcedShutdown [order 1] : [Timer>5]
  SpringTestSetpoint -> ForcedShutdown [order 3] : [Timer>5]
  ForcedShutdown -> SpringTestComplete : [!ECUP_Off]
  data: TestTime output double; ECUP_Off input boolean; DC_Override output int8; Timer input double; LowAdaptError input boolean; TPSHiThresh input boolean; TPSLoThresh input boolean; TimerOld local double; TestComplete output boolean; SetpointMode output int8

== stateflow chart Foreground/Control/VirtualSensors/RawProcessed/APP Sensor Management/Crank APP Lockout ==
  settings: executeAtInitialization=1
  state PassThrough/
    du:APP_Out=APP_In;
    en:APP_Mode=0;
  state OverrideAPP/
    en:APP_Out=0;
    APP_Mode=1;
  (default) -> PassThrough
  PassThrough -> OverrideAPP : [EngineState==3&&EngineState_Old==2&&APP_In>3]
  OverrideAPP -> PassThrough : [APP_In<=3]
  data: APP_Out output double; EngineState input uint8; EngineState_Old input uint8; APP_In input double; APP_Mode output boolean

== matlab function Foreground/Outputs/Coil Control/Coil Enable Manager/EST Enable TDC Counter/EST Enable TDC Counter Stateflow ==
  data: CounterEnabled input double; CounterReset output double; EngineState input double; State input double; ESTEnable output double; StateO output double
function [CounterReset,ESTEnable,StateO] = fcn(CounterEnabled,EngineState,State)

if (State == 0 && CounterEnabled == 1)
    StateO=1;
elseif (State == 1 && EngineState == 2 && CounterEnabled == 1)
    StateO=2;
elseif (State == 2 && EngineState ==3)
    StateO=3;
elseif (State == 2 && EngineState == 1)
    StateO=0;
elseif (State ==3 && EngineState == 1)
    StateO=0;
else
    StateO=State;
end

if State == 0
   ESTEnable=0;
   CounterReset=0;
elseif State == 1
    ESTEnable=1;
    CounterReset=0;
elseif State == 2
    ESTEnable=1;
    CounterReset=1;
elseif State == 3
    ESTEnable=1;
    CounterReset=1;
else
    ESTEnable=0;
    CounterReset=0;
end

== stateflow chart Foreground/Control/Controller/Run/MinGovernor/Idle State Determination/IdleStateMachine ==
  state OffIdle/
    en:IdleState = 3;
  state Flaring/
    en:IdleState = 1;
  state OnIdle/
    en:IdleState = 2;
  (default) -> Flaring
  Flaring -> OffIdle [order 1] : [ThrCmdHigh || !IdleEnable]
  OffIdle -> OnIdle : [IdleEnable && (RPMLow || (RPMInRange && ...
      | ThrCmdLow)) || APPTimeThresh]
  Flaring -> OnIdle [order 2] : [RPMFalling && ThrCmdLow]
  OnIdle -> OffIdle : [!RPMLow&&(ThrCmdHigh || !IdleEnable)]
  data: IdleEnable input boolean; RPMFalling input boolean; RPMInRange input boolean; RPMLow input boolean; ThrCmdLow input boolean; ThrCmdHigh input boolean; IdleState output uint8; APPTimeThresh input boolean

== stateflow chart Foreground/Control/Controller/Run/AirFlow Manager/Power Limit Controller/Power Limiter Setpoint Manager ==
  state NoLimit/
    en:Setpoint=MaxEngPower;
    en:State=0;
  state Level1Limit/
    en:Setpoint=Level1Setpoint;
    en:State=1;
  state Level2Limit/
    en:Setpoint=Level2Setpoint;
    en:State=2;
  state Level3Limit/
    en:Setpoint=Level3Setpoint;
    en:State=3
  state Level4Limit/
    en:Setpoint=Level4Setpoint;
    en:State=4;
  (default) -> NoLimit
  NoLimit -> Level1Limit [order 4] : [Level1]
  Level1Limit -> Level2Limit [order 3] : [Level2]
  Level2Limit -> Level3Limit [order 2] : [Level3]
  Level3Limit -> Level4Limit [order 1] : [Level4]
  NoLimit -> Level2Limit [order 3] : [Level2]
  NoLimit -> Level3Limit [order 2] : [Level3]
  NoLimit -> Level4Limit [order 1] : [Level4]
  Level1Limit -> Level3Limit [order 2] : [Level3]
  Level1Limit -> Level4Limit [order 1] : [Level4]
  Level2Limit -> Level4Limit [order 1] : [Level4]
  Level1Limit -> NoLimit [order 4] : [!Level1&&ETC<PL_ETC]
  Level2Limit -> NoLimit [order 3] : [!Level2&&ETC<PL_ETC]
  Level3Limit -> NoLimit [order 2] : [!Level3&&ETC<PL_ETC]
  Level4Limit -> NoLimit : [!Level4&&ETC<PL_ETC]
  data: State output double; ETC input double; MaxEngPower input double; Level1Setpoint input double; Level2Setpoint input double; Level3Setpoint input double; Level4Setpoint input double; Setpoint output double; Level1 input boolean; Level2 input boolean; Level3 input boolean; Level4 input boolean; PL_ETC input double

== stateflow chart Foreground/Control/VirtualSensors/AirCalcs/Baro Stall State Delay ==
  settings: executeAtInitialization=1
  state Stall/
    en:Enable=1;
  state CrankRun/
    en:Enable=0;
  state StallDelay/
    en:Enable=0;
    en:Timer=0;
    en:TOld=0;
    during:Timer=TOld+dT;
    during:TOld=Timer;
  (default) -> Stall
  Stall -> CrankRun : [EngineState>1]
  CrankRun -> StallDelay : [EngineState==1]
  StallDelay -> Stall [order 2] : [EngineState==1&Timer>TimeThresh]
  StallDelay -> CrankRun [order 1] : [EngineState>1]
  data: EngineState input uint8; TimeThresh input double; dT input double; Timer output double; Enable output boolean; TOld local double
